simulink library "Renault_Library"  (Simulink 8.2)
settings: Solver=ode3  StartTime=0.0  StopTime=10.0  FixedStep=0.020  RelTol=1e-3
undefined symbols (not defined in the file or in its folder): ABC, AssystemProposal, b, chkval, CoeffA_C, CoeffB_C, CoeffMean_C, ConfTime_C, DiagDelay_C, DividePercent, FailureConfActive_C, FallingEdgeDuration_C, HighLevelThresholdVoltage_C, HighLevelVoltage_C, HighLevelVoltageTunable_C, InitValue_C, InputVoltRangeMax_C, InputVoltRangeMin_C, LowActiveContact_C, LowLevelThresholdVoltage_C, LowLevelVoltage_C, MaxOperatingVoltage_C, MinOperatingVoltage_C, mV2V_conversion, OUT1, OutOfRangeHigh_C, OutOfRangeLow_C, PinSupplySaturationMax, PinSupplySaturationMin, Res1Value_C, Res2Value_C, Res3Value_C, RisingEdgeDuration_C, RSA_CANSignalFailMonitoring, SampleTime_C, service14Table, service19Table, service22Table, service24Table, service28Table, service2ETable, service2FTable, service31Table, service87Table, SizeXStateDID2FTable, SizeXTable14, SizeXTable19, SizeXTable22, SizeXTable24, SizeXTable28, SizeXTable2E, SizeXTable2F, SizeXTable31, SizeXTable87, SizeYStateDID2FTable, SizeYTable14, SizeYTable19, SizeYTable22, SizeYTable24, SizeYTable28 ... (+22)
undefined symbols (not defined in the file): BatteryVoltage_ActivationThreshold_C, FrameStatus_AbsentValue_C, FrameStatus_ConfAbsentValue_C, MemThreshold_C, NAMEOFTHEMODEL_SAMPLE_TIME_CST, NbAbs1_C, NbConfAbs1_C, NbConfPres1_C, NbPres1_C, NOMDUSIGNAL_FaultConfTime_C, NOMDUSIGNAL_FaultRehabTime_C, NOMDUSIGNAL_switchvalue_C, NOMDUSIGNAL_unavailablevalue_C, PeriodFrame1_C, ResetTime_C, WakeUpTime_C
libraries: Renault_Library x112, simulink x75
(part 22 of 32)

== system Renault_Library/Services ECU/Diag/RSA_DiagnosticManagement_v02.00/DIAGNOSTIC_SANCTION_TOP/Service19/SubFunction_Supported_Service19 ==
  DIAG_BUFFER_REQUEST : Inport
  BitwiseOperator1 : simulink/Logic and Bit Operations/Bitwise Operator  logicop=AND  UseBitMask=on  BitMask=bin2dec('01111111')  BitMaskRealWorld=Stored Integer
  BitwiseOperator4 : simulink/Logic and Bit Operations/Bitwise Operator  logicop=AND  UseBitMask=on  BitMask=bin2dec('10000000')  BitMaskRealWorld=Stored Integer
  Constant : Constant  OutDataTypeStr=Inherit: Inherit via back propagation  SampleTime=-1
  Constant1 : Constant  Value=6  OutDataTypeStr=Inherit: Inherit via back propagation  SampleTime=-1
  Constant10 : Constant  Value=ReporDTCSnapshotIdentification  OutDataTypeStr=Inherit: Inherit via back propagation  SampleTime=-1
  Constant{5,9,11,13,18,20,23,28,30,34,36,40,56,58,60,62,65,69..74} (23 alike) : Constant  Value=true  OutDataTypeStr=boolean  SampleTime=-1
  Constant12 : Constant  Value=ReportDTCStoredDataByRecordNumber  OutDataTypeStr=Inherit: Inherit via back propagation  SampleTime=-1
  Constant14 : Constant  Value=5  OutDataTypeStr=Inherit: Inherit via back propagation  SampleTime=-1
  Constant15 : Constant  Value=ReportSupportedDTC  OutDataTypeStr=Inherit: Inherit via back propagation  SampleTime=-1
  Constant16 : Constant  OutDataTypeStr=Inherit: Inherit via back propagation  SampleTime=-1
  Constant17 : Constant  Value=ReporDTCSnapshotRecordByDTCNumber  OutDataTypeStr=Inherit: Inherit via back propagation  SampleTime=-1
  Constant19 : Constant  Value=4  OutDataTypeStr=Inherit: Inherit via back propagation  SampleTime=-1
  Constant2 : Constant  Value=2  OutDataTypeStr=Inherit: Inherit via back propagation  SampleTime=-1
  Constant21 : Constant  Value=10  OutDataTypeStr=Inherit: Inherit via back propagation  SampleTime=-1
  Constant22 : Constant  Value=ReportSeverityInformationOfDTC  OutDataTypeStr=Inherit: Inherit via back propagation  SampleTime=-1
  Constant24 : Constant  Value=9  OutDataTypeStr=Inherit: Inherit via back propagation  SampleTime=-1
  Constant25 : Constant  Value=7  OutDataTypeStr=Inherit: Inherit via back propagation  SampleTime=-1
  Constant26 : Constant  Value=ReportDTCExtendedDataRecordByDTCNumber  OutDataTypeStr=Inherit: Inherit via back propagation  SampleTime=-1
  Constant27 : Constant  Value=8  OutDataTypeStr=Inherit: Inherit via back propagation  SampleTime=-1
  Constant29 : Constant  Value=ReportNumberOfDTCBySeverityMaskRecord  OutDataTypeStr=Inherit: Inherit via back propagation  SampleTime=-1
  Constant3 : Constant  Value=ReportNumberOfDTCByStatusMask  OutDataTypeStr=Inherit: Inherit via back propagation  SampleTime=-1
  Constant31 : Constant  Value=11  OutDataTypeStr=Inherit: Inherit via back propagation  SampleTime=-1
  Constant32 : Constant  Value=16  OutDataTypeStr=Inherit: Inherit via back propagation  SampleTime=-1
  Constant33 : Constant  Value=ReportMostRecentTestFailedDTC  OutDataTypeStr=Inherit: Inherit via back propagation  SampleTime=-1
  Constant35 : Constant  Value=ReportMirrorMemoryDTCByStatusMask  OutDataTypeStr=Inherit: Inherit via back propagation  SampleTime=-1
  Constant37 : Constant  Value=15  OutDataTypeStr=Inherit: Inherit via back propagation  SampleTime=-1
  Constant38 : Constant  Value=ReportDTCFaultDetectionCounter  OutDataTypeStr=Inherit: Inherit via back propagation  SampleTime=-1
  Constant39 : Constant  Value=ReportMostRecentConfimedDTC  OutDataTypeStr=Inherit: Inherit via back propagation  SampleTime=-1
  Constant4 : Constant  Value=3  OutDataTypeStr=Inherit: Inherit via back propagation  SampleTime=-1
  Constant41 : Constant  Value=14  OutDataTypeStr=Inherit: Inherit via back propagation  SampleTime=-1
  Constant42 : Constant  Value=12  OutDataTypeStr=Inherit: Inherit via back propagation  SampleTime=-1
  Constant44 : Constant  Value=20  OutDataTypeStr=Inherit: Inherit via back propagation  SampleTime=-1
  Constant45 : Constant  Value=ReportEmissionsRelatedOBDDTCByStatusMask  OutDataTypeStr=Inherit: Inherit via back propagation  SampleTime=-1
  Constant47 : Constant  Value=19  OutDataTypeStr=Inherit: Inherit via back propagation  SampleTime=-1
  Constant48 : Constant  Value=17  OutDataTypeStr=Inherit: Inherit via back propagation  SampleTime=-1
  Constant49 : Constant  Value=ReportMirrorMemoryDTCExtendedDataRecordByDTCNumber  OutDataTypeStr=Inherit: Inherit via back propagation  SampleTime=-1
  Constant50 : Constant  Value=18  OutDataTypeStr=Inherit: Inherit via back propagation  SampleTime=-1
  Constant52 : Constant  Value=ReportNumberOfMirrorMemoryDTCByStatusMask  OutDataTypeStr=Inherit: Inherit via back propagation  SampleTime=-1
  Constant53 : Constant  Value=ReportFirstTestFailedDTC  OutDataTypeStr=Inherit: Inherit via back propagation  SampleTime=-1
  Constant55 : Constant  Value=13  OutDataTypeStr=Inherit: Inherit via back propagation  SampleTime=-1
  Constant57 : Constant  Value=ReportNumberOfEmissionsRelatedOBDDTCByStatusMask  OutDataTypeStr=Inherit: Inherit via back propagation  SampleTime=-1
  Constant59 : Constant  Value=ReportFirstConfirmedDTC  OutDataTypeStr=Inherit: Inherit via back propagation  SampleTime=-1
  Constant6 : Constant  Value=ReportDTCBySeverityMaskRecord  OutDataTypeStr=Inherit: Inherit via back propagation  SampleTime=-1
  Constant61 : Constant  Value=ReportWWHOBDDTCWithPermanentStatus  OutDataTypeStr=Inherit: Inherit via back propagation  SampleTime=-1
  Constant63 : Constant  Value=85  OutDataTypeStr=Inherit: Inherit via back propagation  SampleTime=-1
  Constant64 : Constant  Value=ReportWWHOBDDTCByMaskRecord  OutDataTypeStr=Inherit: Inherit via back propagation  SampleTime=-1
  Constant66 : Constant  Value=66  OutDataTypeStr=Inherit: Inherit via back propagation  SampleTime=-1
  Constant67 : Constant  Value=21  OutDataTypeStr=Inherit: Inherit via back propagation  SampleTime=-1
  Constant68 : Constant  Value=ReportDTCWithPermanetStatus  OutDataTypeStr=Inherit: Inherit via back propagation  SampleTime=-1
  Constant8 : Constant  Value=ReportDTCByStatusMask  OutDataTypeStr=Inherit: Inherit via back propagation  SampleTime=-1
  LogicalOperator{,1..3,5..23} (23 alike) : Logic  OutDataTypeStr=boolean
  LogicalOperator4 : Logic  Operator=OR  Inputs=23  OutDataTypeStr=boolean
  RelationalOperator{,1..46} (47 alike) : RelationalOperator  Operator===  OutDataTypeStr=boolean  InputSameDT=on
  Selector : Selector  InputPortWidth=4095  IndexOptions=Index vector (dialog)  Indices=[2]  OutputSizes=1
  ShiftArithmetic3 : ArithShift  BitShiftNumber=7
  SUBFUNCTION_SUPPORTED : Outport
  SUPPRESS_POS_RES : Outport  Port=2
  wires:
  DIAG_BUFFER_REQUEST/1 -> Selector/1
  Constant/1 -> RelationalOperator/2
  Constant2/1 -> RelationalOperator2/2
  Constant4/1 -> RelationalOperator3/2
  Constant3/1 -> RelationalOperator4/1
  Constant5/1 -> RelationalOperator4/2
  RelationalOperator/1 -> LogicalOperator/2
  RelationalOperator4/1 -> LogicalOperator/1
  Constant36/1 -> RelationalOperator6/2
  Constant8/1 -> RelationalOperator6/1
  RelationalOperator2/1 -> LogicalOperator2/2
  RelationalOperator6/1 -> LogicalOperator2/1
  Constant10/1 -> RelationalOperator7/1
  Constant56/1 -> RelationalOperator7/2
  RelationalOperator7/1 -> LogicalOperator3/1
  RelationalOperator3/1 -> LogicalOperator3/2
  RelationalOperator16/1 -> SUPPRESS_POS_RES/1
  BitwiseOperator4/1 -> ShiftArithmetic3/1
  ShiftArithmetic3/1 -> RelationalOperator16/1
  Selector/1 -> BitwiseOperator4/1, BitwiseOperator1/1
  Constant16/1 -> RelationalOperator16/2
  BitwiseOperator1/1 -> RelationalOperator/1, RelationalOperator2/1, RelationalOperator3/1, RelationalOperator12/1, RelationalOperator8/1, RelationalOperator1/1, RelationalOperator11/1, RelationalOperator14/1, RelationalOperator5/1, RelationalOperator19/1, RelationalOperator21/1, RelationalOperator32/1, RelationalOperator34/1, RelationalOperator25/1, RelationalOperator39/1, RelationalOperator22/1, RelationalOperator24/1, RelationalOperator27/1, RelationalOperator36/1, RelationalOperator31/1, RelationalOperator42/1, RelationalOperator46/1, RelationalOperator44/1
  Constant19/1 -> RelationalOperator12/2
  Constant17/1 -> RelationalOperator13/1
  Constant58/1 -> RelationalOperator13/2
  RelationalOperator13/1 -> LogicalOperator6/1
  RelationalOperator12/1 -> LogicalOperator6/2
  Constant14/1 -> RelationalOperator8/2
  Constant12/1 -> RelationalOperator9/1
  Constant71/1 -> RelationalOperator9/2
  RelationalOperator9/1 -> LogicalOperator5/1
  RelationalOperator8/1 -> LogicalOperator5/2
  Constant1/1 -> RelationalOperator1/2
  Constant25/1 -> RelationalOperator11/2
  Constant27/1 -> RelationalOperator14/2
  Constant60/1 -> RelationalOperator15/2
  RelationalOperator1/1 -> LogicalOperator1/2
  RelationalOperator15/1 -> LogicalOperator1/1
  Constant62/1 -> RelationalOperator17/2
  Constant29/1 -> RelationalOperator17/1
  RelationalOperator11/1 -> LogicalOperator7/2
  RelationalOperator17/1 -> LogicalOperator7/1
  Constant6/1 -> RelationalOperator18/1
  Constant65/1 -> RelationalOperator18/2
  RelationalOperator18/1 -> LogicalOperator8/1
  RelationalOperator14/1 -> LogicalOperator8/2
  Constant24/1 -> RelationalOperator5/2
  Constant22/1 -> RelationalOperator10/1
  Constant69/1 -> RelationalOperator10/2
  RelationalOperator10/1 -> LogicalOperator10/1
  RelationalOperator5/1 -> LogicalOperator10/2
  Constant21/1 -> RelationalOperator19/2
  Constant15/1 -> RelationalOperator20/1
  Constant70/1 -> RelationalOperator20/2
  RelationalOperator20/1 -> LogicalOperator9/1
  RelationalOperator19/1 -> LogicalOperator9/2
  Constant31/1 -> RelationalOperator21/2
  Constant42/1 -> RelationalOperator32/2
  Constant55/1 -> RelationalOperator34/2
  Constant53/1 -> RelationalOperator35/1
  Constant72/1 -> RelationalOperator35/2
  RelationalOperator21/1 -> LogicalOperator11/2
  RelationalOperator35/1 -> LogicalOperator11/1
  Constant73/1 -> RelationalOperator37/2
  Constant59/1 -> RelationalOperator37/1
  RelationalOperator32/1 -> LogicalOperator14/2
  RelationalOperator37/1 -> LogicalOperator14/1
  Constant33/1 -> RelationalOperator38/1
  Constant11/1 -> RelationalOperator38/2
  RelationalOperator38/1 -> LogicalOperator15/1
  RelationalOperator34/1 -> LogicalOperator15/2
  Constant41/1 -> RelationalOperator25/2
  Constant39/1 -> RelationalOperator26/1
  Constant9/1 -> RelationalOperator26/2
  RelationalOperator26/1 -> LogicalOperator17/1
  RelationalOperator25/1 -> LogicalOperator17/2
  Constant37/1 -> RelationalOperator39/2
  Constant35/1 -> RelationalOperator40/1
  Constant13/1 -> RelationalOperator40/2
  RelationalOperator40/1 -> LogicalOperator16/1
  RelationalOperator39/1 -> LogicalOperator16/2
  Constant32/1 -> RelationalOperator22/2
  Constant48/1 -> RelationalOperator24/2
  Constant50/1 -> RelationalOperator27/2
  Constant18/1 -> RelationalOperator28/2
  RelationalOperator22/1 -> LogicalOperator12/2
  RelationalOperator28/1 -> LogicalOperator12/1
  Constant20/1 -> RelationalOperator29/2
  Constant52/1 -> RelationalOperator29/1
  RelationalOperator24/1 -> LogicalOperator18/2
  RelationalOperator29/1 -> LogicalOperator18/1
  Constant57/1 -> RelationalOperator30/1
  Constant23/1 -> RelationalOperator30/2
  RelationalOperator30/1 -> LogicalOperator19/1
  RelationalOperator27/1 -> LogicalOperator19/2
  Constant47/1 -> RelationalOperator36/2
  Constant45/1 -> RelationalOperator23/1
  Constant74/1 -> RelationalOperator23/2
  RelationalOperator23/1 -> LogicalOperator13/1
  RelationalOperator36/1 -> LogicalOperator13/2
  Constant38/1 -> RelationalOperator33/1
  Constant28/1 -> RelationalOperator33/2
  RelationalOperator33/1 -> LogicalOperator20/1
  RelationalOperator31/1 -> LogicalOperator20/2
  Constant44/1 -> RelationalOperator31/2
  Constant67/1 -> RelationalOperator42/2
  Constant68/1 -> RelationalOperator43/1
  Constant30/1 -> RelationalOperator43/2
  RelationalOperator43/1 -> LogicalOperator22/1
  RelationalOperator42/1 -> LogicalOperator22/2
  Constant66/1 -> RelationalOperator46/2
  Constant64/1 -> RelationalOperator41/1
  Constant34/1 -> RelationalOperator41/2
  RelationalOperator41/1 -> LogicalOperator21/1
  RelationalOperator46/1 -> LogicalOperator21/2
  Constant61/1 -> RelationalOperator45/1
  Constant40/1 -> RelationalOperator45/2
  RelationalOperator45/1 -> LogicalOperator23/1
  RelationalOperator44/1 -> LogicalOperator23/2
  Constant63/1 -> RelationalOperator44/2
  LogicalOperator/1 -> LogicalOperator4/1
  LogicalOperator2/1 -> LogicalOperator4/2
  LogicalOperator3/1 -> LogicalOperator4/3
  LogicalOperator6/1 -> LogicalOperator4/4
  LogicalOperator5/1 -> LogicalOperator4/5
  LogicalOperator1/1 -> LogicalOperator4/6
  LogicalOperator7/1 -> LogicalOperator4/7
  LogicalOperator8/1 -> LogicalOperator4/8
  LogicalOperator10/1 -> LogicalOperator4/9
  LogicalOperator9/1 -> LogicalOperator4/10
  LogicalOperator11/1 -> LogicalOperator4/11
  LogicalOperator14/1 -> LogicalOperator4/12
  LogicalOperator15/1 -> LogicalOperator4/13
  LogicalOperator17/1 -> LogicalOperator4/14
  LogicalOperator16/1 -> LogicalOperator4/15
  LogicalOperator12/1 -> LogicalOperator4/16
  LogicalOperator18/1 -> LogicalOperator4/17
  LogicalOperator19/1 -> LogicalOperator4/18
  LogicalOperator13/1 -> LogicalOperator4/19
  LogicalOperator20/1 -> LogicalOperator4/20
  LogicalOperator22/1 -> LogicalOperator4/21
  LogicalOperator21/1 -> LogicalOperator4/22
  LogicalOperator23/1 -> LogicalOperator4/23
  Constant26/1 -> RelationalOperator15/1
  Constant49/1 -> RelationalOperator28/1
  LogicalOperator4/1 -> SUBFUNCTION_SUPPORTED/1

== system Renault_Library/Services ECU/Diag/RSA_DiagnosticManagement_v02.00/DIAGNOSTIC_SANCTION_TOP/Service22/Check_Lenght_Message_Response_Service22 ==
  RESPONSE_SIZE : Inport
  Constant8 : Constant  Value=4094  OutDataTypeStr=Inherit: Inherit via back propagation  SampleTime=-1
  RelationalOperator9 : RelationalOperator  Operator=<  OutDataTypeStr=boolean  InputSameDT=on
  LENGTH_RESPONSE_CORRECT : Outport
  wires:
  RESPONSE_SIZE/1 -> RelationalOperator9/1
  Constant8/1 -> RelationalOperator9/2
  RelationalOperator9/1 -> LENGTH_RESPONSE_CORRECT/1

== system Renault_Library/Services ECU/Diag/RSA_DiagnosticManagement_v02.00/DIAGNOSTIC_SANCTION_TOP/Service22/Check_Lenght_Message_Service22 ==
  REQ_DATA_NUMBER : Inport
  Constant1 : Constant  Value=3  OutDataTypeStr=Inherit: Inherit via back propagation  SampleTime=-1
  Constant8 : Constant  Value=4094  OutDataTypeStr=Inherit: Inherit via back propagation  SampleTime=-1
  LogicalOperator : Logic  OutDataTypeStr=boolean
  RelationalOperator1 : RelationalOperator  OutDataTypeStr=boolean  InputSameDT=on
  RelationalOperator9 : RelationalOperator  Operator=<  OutDataTypeStr=boolean  InputSameDT=on
  LENGTH_MESSAGE_CORRECT : Outport
  wires:
  REQ_DATA_NUMBER/1 -> RelationalOperator9/1, RelationalOperator1/1
  Constant8/1 -> RelationalOperator9/2
  Constant1/1 -> RelationalOperator1/2
  RelationalOperator9/1 -> LogicalOperator/1
  RelationalOperator1/1 -> LogicalOperator/2
  LogicalOperator/1 -> LENGTH_MESSAGE_CORRECT/1

== system Renault_Library/Services ECU/Diag/RSA_DiagnosticManagement_v02.00/DIAGNOSTIC_SANCTION_TOP/Service22/Parameter_Valid_Service22 ==
  REQ_DATA_NUMBER : Inport
  DIAG_BUFFER_REQUEST : Inport  Port=2
  SESSION_ACTIVE : Inport  Port=3
  Parameter_service22 : Stateflow chart  TreatAsAtomicUnit=on
  RESPONSE_SIZE : Outport
  SECURITY_ACCESS_ACCEPTED : Outport  Port=2
  PARAMETER_VALID : Outport  Port=3
  LIST_DID_SERVICE_22 : Outport  Port=4  PortDimensions=[2046 3]
  wires:
  DIAG_BUFFER_REQUEST/1 -> Parameter_service22/1(DIAG_BUFFER_REQUEST)
  REQ_DATA_NUMBER/1 -> Parameter_service22/2(REQ_DATA_NUMBER)
  Parameter_service22/1(PARAMETER_VALID) -> PARAMETER_VALID/1
  Parameter_service22/2(LIST_DID_SERVICE_22) -> LIST_DID_SERVICE_22/1
  Parameter_service22/3(RESPONSE_SIZE) -> RESPONSE_SIZE/1
  Parameter_service22/4(SECURITY_ACCESS_ACCEPTED) -> SECURITY_ACCESS_ACCEPTED/1
  SESSION_ACTIVE/1 -> Parameter_service22/3(SESSION_ACTIVE)

== system Renault_Library/Services ECU/Diag/RSA_DiagnosticManagement_v02.00/DIAGNOSTIC_SANCTION_TOP/Service23/Check_Lenght_Message_Service23 ==
  REQ_DATA_NUMBER : Inport
  Constant1 : Constant  Value=4  OutDataTypeStr=Inherit: Inherit via back propagation  SampleTime=-1
  Constant8 : Constant  Value=4094  OutDataTypeStr=Inherit: Inherit via back propagation  SampleTime=-1
  LogicalOperator : Logic  OutDataTypeStr=boolean
  RelationalOperator1 : RelationalOperator  OutDataTypeStr=boolean  InputSameDT=on
  RelationalOperator9 : RelationalOperator  Operator=<  OutDataTypeStr=boolean  InputSameDT=on
  LENGTH_MESSAGE_CORRECT : Outport
  wires:
  REQ_DATA_NUMBER/1 -> RelationalOperator9/1, RelationalOperator1/1
  Constant8/1 -> RelationalOperator9/2
  Constant1/1 -> RelationalOperator1/2
  RelationalOperator9/1 -> LogicalOperator/1
  RelationalOperator1/1 -> LogicalOperator/2
  LogicalOperator/1 -> LENGTH_MESSAGE_CORRECT/1

== system Renault_Library/Services ECU/Diag/RSA_DiagnosticManagement_v02.00/DIAGNOSTIC_SANCTION_TOP/Service23/Parameter_Valid_Service23 ==
  REQ_DATA_NUMBER : Inport
  DIAG_BUFFER_REQUEST : Inport  Port=2
  SESSION_ACTIVE : Inport  Port=3
  Parameter_service23 : Stateflow chart  TreatAsAtomicUnit=on
  SECURITY_ACCESS_ACCEPTED : Outport
  PARAMETER_VALID : Outport  Port=2
  wires:
  DIAG_BUFFER_REQUEST/1 -> Parameter_service23/1(DIAG_BUFFER_REQUEST)
  REQ_DATA_NUMBER/1 -> Parameter_service23/2(REQ_DATA_NUMBER)
  Parameter_service23/1(PARAMETER_VALID) -> PARAMETER_VALID/1
  Parameter_service23/2(SECURITY_ACCESS_ACCEPTED) -> SECURITY_ACCESS_ACCEPTED/1
  SESSION_ACTIVE/1 -> Parameter_service23/3(SESSION_ACTIVE)

== system Renault_Library/Services ECU/Diag/RSA_DiagnosticManagement_v02.00/DIAGNOSTIC_SANCTION_TOP/Service24/Check_Lenght_Message_Service24 ==
  REQ_DATA_NUMBER : Inport
  Constant8 : Constant  Value=3  OutDataTypeStr=Inherit: Inherit via back propagation  SampleTime=-1
  RelationalOperator9 : RelationalOperator  Operator===  OutDataTypeStr=boolean  InputSameDT=on
  LENGTH_MESSAGE_CORRECT : Outport
  wires:
  REQ_DATA_NUMBER/1 -> RelationalOperator9/1
  Constant8/1 -> RelationalOperator9/2
  RelationalOperator9/1 -> LENGTH_MESSAGE_CORRECT/1

== system Renault_Library/Services ECU/Diag/RSA_DiagnosticManagement_v02.00/DIAGNOSTIC_SANCTION_TOP/Service24/Parameter_Valid_Service24 ==
  DIAG_BUFFER_REQUEST : Inport
  SESSION_ACTIVE : Inport  Port=2
  Parameter_service24 : Stateflow chart  TreatAsAtomicUnit=on
  SECURITY_ACCESS_ACCEPTED : Outport
  PARAMETER_VALID : Outport  Port=2
  wires:
  DIAG_BUFFER_REQUEST/1 -> Parameter_service24/1(DIAG_BUFFER_REQUEST)
  Parameter_service24/1(PARAMETER_VALID) -> PARAMETER_VALID/1
  Parameter_service24/2(SECURITY_ACCESS_ACCEPTED) -> SECURITY_ACCESS_ACCEPTED/1
  SESSION_ACTIVE/1 -> Parameter_service24/2(SESSION_ACTIVE)

== system Renault_Library/Services ECU/Diag/RSA_DiagnosticManagement_v02.00/DIAGNOSTIC_SANCTION_TOP/Service27/Check_Lenght_Message_Service27 ==
  REQ_DATA_NUMBER : Inport
  Constant1 : Constant  Value=2  OutDataTypeStr=Inherit: Inherit via back propagation  SampleTime=-1
  Constant8 : Constant  Value=4094  OutDataTypeStr=Inherit: Inherit via back propagation  SampleTime=-1
  LogicalOperator : Logic  OutDataTypeStr=boolean
  RelationalOperator1 : RelationalOperator  OutDataTypeStr=boolean  InputSameDT=on
  RelationalOperator9 : RelationalOperator  Operator=<  OutDataTypeStr=boolean  InputSameDT=on
  LENGTH_MESSAGE_CORRECT : Outport
  wires:
  REQ_DATA_NUMBER/1 -> RelationalOperator9/1, RelationalOperator1/1
  Constant8/1 -> RelationalOperator9/2
  Constant1/1 -> RelationalOperator1/2
  RelationalOperator9/1 -> LogicalOperator/1
  RelationalOperator1/1 -> LogicalOperator/2
  LogicalOperator/1 -> LENGTH_MESSAGE_CORRECT/1

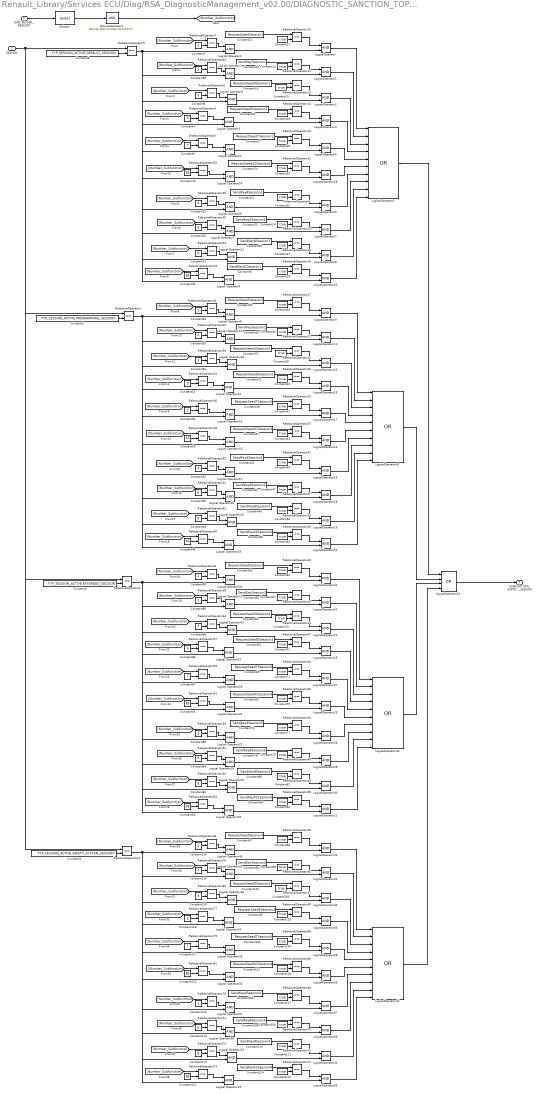
== system Renault_Library/Services ECU/Diag/RSA_DiagnosticManagement_v02.00/DIAGNOSTIC_SANCTION_TOP/Service27/SubFunction_In_Active_Session_Service27 ==
  SESSION : Inport
  DIAG_BUFFER_REQUEST : Inport  Port=2
  BitwiseOperator1 : simulink/Logic and Bit Operations/Bitwise Operator  logicop=AND  UseBitMask=on  BitMask=bin2dec('01111111')  BitMaskRealWorld=Stored Integer
  Constant1 : Constant  Value=TYP_SESSION_ACTIVE.PROGRAMMING_SESSION  OutDataTypeStr=Inherit: Inherit via back propagation  SampleTime=-1
  Constant10 : Constant  Value=RequestSeed5Session1  OutDataTypeStr=Inherit: Inherit via back propagation  SampleTime=-1
  Constant{11,13,15,18..20,22,24,27..28,34,36,39..41,43,45,47,58,62,64,67,70..72,75,77,79,81,92,96,98,100,103..105,107,109,111,122} (40 alike) : Constant  Value=true  OutDataTypeStr=boolean  SampleTime=-1
  Constant101 : Constant  Value=RequestSeed3Session4  OutDataTypeStr=Inherit: Inherit via back propagation  SampleTime=-1
  Constant102 : Constant  Value=RequestSeed7Session4  OutDataTypeStr=Inherit: Inherit via back propagation  SampleTime=-1
  Constant106 : Constant  Value=SendKey4Session4  OutDataTypeStr=Inherit: Inherit via back propagation  SampleTime=-1
  Constant108 : Constant  Value=SendKey6Session4  OutDataTypeStr=Inherit: Inherit via back propagation  SampleTime=-1
  Constant110 : Constant  Value=SendKey8Session4  OutDataTypeStr=Inherit: Inherit via back propagation  SampleTime=-1
  Constant{29,48,82,112} (4 alike) : Constant  Value=66  OutDataTypeStr=Inherit: Inherit via back propagation  SampleTime=-1
  Constant{31,49,83,113} (4 alike) : Constant  Value=8  OutDataTypeStr=Inherit: Inherit via back propagation  SampleTime=-1
  Constant{32,50,84,114} (4 alike) : Constant  Value=6  OutDataTypeStr=Inherit: Inherit via back propagation  SampleTime=-1
  Constant{33,51,85,115} (4 alike) : Constant  Value=4  OutDataTypeStr=Inherit: Inherit via back propagation  SampleTime=-1
  Constant{4,52,86,116} (4 alike) : Constant  Value=5  OutDataTypeStr=Inherit: Inherit via back propagation  SampleTime=-1
  Constant{5,53,87,117} (4 alike) : Constant  Value=7  OutDataTypeStr=Inherit: Inherit via back propagation  SampleTime=-1
  Constant{6,54,88,118} (4 alike) : Constant  Value=3  OutDataTypeStr=Inherit: Inherit via back propagation  SampleTime=-1
  Constant{55,65,89,119} (4 alike) : Constant  Value=2  OutDataTypeStr=Inherit: Inherit via back propagation  SampleTime=-1
  Constant12 : Constant  Value=RequestSeedSession1  OutDataTypeStr=Inherit: Inherit via back propagation  SampleTime=-1
  Constant{7,56,90,120} (4 alike) : Constant  OutDataTypeStr=Inherit: Inherit via back propagation  SampleTime=-1
  Constant121 : Constant  Value=RequestSeed41Session4  OutDataTypeStr=Inherit: Inherit via back propagation  SampleTime=-1
  Constant{59,76,93,123} (4 alike) : Constant  Value=65  OutDataTypeStr=Inherit: Inherit via back propagation  SampleTime=-1
  Constant124 : Constant  Value=SendKey42Session4  OutDataTypeStr=Inherit: Inherit via back propagation  SampleTime=-1
  Constant14 : Constant  Value=SendKeySession1  OutDataTypeStr=Inherit: Inherit via back propagation  SampleTime=-1
  Constant16 : Constant  Value=RequestSeed3Session1  OutDataTypeStr=Inherit: Inherit via back propagation  SampleTime=-1
  Constant17 : Constant  Value=RequestSeed7Session1  OutDataTypeStr=Inherit: Inherit via back propagation  SampleTime=-1
  Constant2 : Constant  Value=TYP_SESSION_ACTIVE.EXTENDED_SESSION  OutDataTypeStr=Inherit: Inherit via back propagation  SampleTime=-1
  Constant21 : Constant  Value=SendKey4Session1  OutDataTypeStr=Inherit: Inherit via back propagation  SampleTime=-1
  Constant23 : Constant  Value=SendKey6Session1  OutDataTypeStr=Inherit: Inherit via back propagation  SampleTime=-1
  Constant25 : Constant  Value=SendKey8Session1  OutDataTypeStr=Inherit: Inherit via back propagation  SampleTime=-1
  Constant26 : Constant  Value=RequestSeed5Session2  OutDataTypeStr=Inherit: Inherit via back propagation  SampleTime=-1
  Constant3 : Constant  Value=TYP_SESSION_ACTIVE.SAFETY_SYSTEM_SESSION  OutDataTypeStr=Inherit: Inherit via back propagation  SampleTime=-1
  Constant30 : Constant  Value=RequestSeedSession2  OutDataTypeStr=Inherit: Inherit via back propagation  SampleTime=-1
  Constant35 : Constant  Value=SendKeySession2  OutDataTypeStr=Inherit: Inherit via back propagation  SampleTime=-1
  Constant37 : Constant  Value=RequestSeed3Session2  OutDataTypeStr=Inherit: Inherit via back propagation  SampleTime=-1
  Constant38 : Constant  Value=RequestSeed7Session2  OutDataTypeStr=Inherit: Inherit via back propagation  SampleTime=-1
  Constant42 : Constant  Value=SendKey4Session2  OutDataTypeStr=Inherit: Inherit via back propagation  SampleTime=-1
  Constant44 : Constant  Value=SendKey6Session2  OutDataTypeStr=Inherit: Inherit via back propagation  SampleTime=-1
  Constant46 : Constant  Value=SendKey8Session2  OutDataTypeStr=Inherit: Inherit via back propagation  SampleTime=-1
  Constant57 : Constant  Value=RequestSeed41Session2  OutDataTypeStr=Inherit: Inherit via back propagation  SampleTime=-1
  Constant60 : Constant  Value=SendKey42Session2  OutDataTypeStr=Inherit: Inherit via back propagation  SampleTime=-1
  Constant61 : Constant  Value=RequestSeed5Session3  OutDataTypeStr=Inherit: Inherit via back propagation  SampleTime=-1
  Constant63 : Constant  Value=RequestSeedSession3  OutDataTypeStr=Inherit: Inherit via back propagation  SampleTime=-1
  Constant66 : Constant  Value=SendKeySession3  OutDataTypeStr=Inherit: Inherit via back propagation  SampleTime=-1
  Constant68 : Constant  Value=RequestSeed3Session3  OutDataTypeStr=Inherit: Inherit via back propagation  SampleTime=-1
  Constant69 : Constant  Value=RequestSeed7Session3  OutDataTypeStr=Inherit: Inherit via back propagation  SampleTime=-1
  Constant73 : Constant  Value=SendKey4Session3  OutDataTypeStr=Inherit: Inherit via back propagation  SampleTime=-1
  Constant74 : Constant  Value=RequestSeed41Session1  OutDataTypeStr=Inherit: Inherit via back propagation  SampleTime=-1
  Constant78 : Constant  Value=SendKey6Session3  OutDataTypeStr=Inherit: Inherit via back propagation  SampleTime=-1
  Constant8 : Constant  Value=TYP_SESSION_ACTIVE.DEFAULT_SESSION  OutDataTypeStr=Inherit: Inherit via back propagation  SampleTime=-1
  Constant80 : Constant  Value=SendKey8Session3  OutDataTypeStr=Inherit: Inherit via back propagation  SampleTime=-1
  Constant9 : Constant  Value=SendKey42Session1  OutDataTypeStr=Inherit: Inherit via back propagation  SampleTime=-1
  Constant91 : Constant  Value=RequestSeed41Session3  OutDataTypeStr=Inherit: Inherit via back propagation  SampleTime=-1
  Constant94 : Constant  Value=SendKey42Session3  OutDataTypeStr=Inherit: Inherit via back propagation  SampleTime=-1
  Constant95 : Constant  Value=RequestSeed5Session4  OutDataTypeStr=Inherit: Inherit via back propagation  SampleTime=-1
  Constant97 : Constant  Value=RequestSeedSession4  OutDataTypeStr=Inherit: Inherit via back propagation  SampleTime=-1
  Constant99 : Constant  Value=SendKeySession4  OutDataTypeStr=Inherit: Inherit via back propagation  SampleTime=-1
  Logical Operator{1..40} (40 alike) : Logic  OutDataTypeStr=boolean
  LogicalOperator{,1..3,5..23,25..41} (40 alike) : Logic  OutDataTypeStr=boolean
  LogicalOperator24 : Logic  Operator=OR  Inputs=4  OutDataTypeStr=boolean
  LogicalOperator{4,42..44} (4 alike) : Logic  Operator=OR  Inputs=10  OutDataTypeStr=boolean
  RelationalOperator{1..84} (84 alike) : RelationalOperator  Operator===  OutDataTypeStr=boolean  InputSameDT=on
  Selector : Selector  InputPortWidth=4095  IndexOptions=Index vector (dialog)  Indices=[2]  OutputSizes=1
  SUBFUNCTION_SUPPORTED_IN_ACTIVE_SESSION : Outport
  wires:
  Constant8/1 -> RelationalOperator9/2
  SESSION/1 -> RelationalOperator9/1, RelationalOperator1/1, RelationalOperator2/1, RelationalOperator3/1
  Constant1/1 -> RelationalOperator1/2
  Constant2/1 -> RelationalOperator2/2
  Constant3/1 -> RelationalOperator3/2
  Selector/1 -> BitwiseOperator1/1
  DIAG_BUFFER_REQUEST/1 -> Selector/1
  Constant7/1 -> RelationalOperator7/2
  Constant12/1 -> RelationalOperator10/1
  Constant11/1 -> RelationalOperator10/2
  RelationalOperator10/1 -> LogicalOperator/1
  Constant13/1 -> RelationalOperator11/2
  Constant14/1 -> RelationalOperator11/1
  RelationalOperator11/1 -> LogicalOperator1/1
  Constant15/1 -> RelationalOperator12/2
  Constant16/1 -> RelationalOperator12/1
  RelationalOperator12/1 -> LogicalOperator2/1
  Constant10/1 -> RelationalOperator14/1
  Constant18/1 -> RelationalOperator14/2
  RelationalOperator14/1 -> LogicalOperator3/1
  Constant17/1 -> RelationalOperator13/1
  Constant19/1 -> RelationalOperator13/2
  RelationalOperator13/1 -> LogicalOperator6/1
  Logical Operator2/1 -> LogicalOperator1/2
  Logical Operator1/1 -> LogicalOperator/2
  RelationalOperator9/1 -> Logical Operator1/2, Logical Operator2/2, Logical Operator3/2, Logical Operator4/2, Logical Operator5/2, Logical Operator24/2, Logical Operator6/2, Logical Operator7/2, Logical Operator11/2, Logical Operator9/2
  Constant65/1 -> RelationalOperator45/2
  RelationalOperator45/1 -> Logical Operator2/1
  Constant4/1 -> RelationalOperator4/2
  Constant5/1 -> RelationalOperator5/2
  RelationalOperator5/1 -> Logical Operator4/1
  Logical Operator4/1 -> LogicalOperator6/2
  Logical Operator3/1 -> LogicalOperator3/2
  Constant6/1 -> RelationalOperator6/2
  Logical Operator5/1 -> LogicalOperator2/2
  RelationalOperator7/1 -> Logical Operator1/1
  RelationalOperator4/1 -> Logical Operator3/1
  RelationalOperator6/1 -> Logical Operator5/1
  LogicalOperator24/1 -> SUBFUNCTION_SUPPORTED_IN_ACTIVE_SESSION/1
  BitwiseOperator1/1 -> [Number_Subfunction]
  [Number_Subfunction] -> RelationalOperator7/1
  [Number_Subfunction] -> RelationalOperator45/1
  [Number_Subfunction] -> RelationalOperator6/1
  [Number_Subfunction] -> RelationalOperator4/1
  [Number_Subfunction] -> RelationalOperator5/1
  Constant74/1 -> RelationalOperator51/1
  Constant20/1 -> RelationalOperator51/2
  RelationalOperator51/1 -> LogicalOperator28/1
  Constant76/1 -> RelationalOperator52/2
  RelationalOperator52/1 -> Logical Operator24/1
  Logical Operator24/1 -> LogicalOperator28/2
  [Number_Subfunction] -> RelationalOperator52/1
  Constant33/1 -> RelationalOperator25/2
  Constant21/1 -> RelationalOperator8/1
  Constant22/1 -> RelationalOperator8/2
  RelationalOperator8/1 -> LogicalOperator5/1
  Constant24/1 -> RelationalOperator15/2
  Constant23/1 -> RelationalOperator15/1
  RelationalOperator15/1 -> LogicalOperator7/1
  Constant27/1 -> RelationalOperator16/2
  Constant25/1 -> RelationalOperator16/1
  RelationalOperator16/1 -> LogicalOperator8/1
  Constant9/1 -> RelationalOperator18/1
  Constant28/1 -> RelationalOperator18/2
  RelationalOperator18/1 -> LogicalOperator10/1
  Logical Operator7/1 -> LogicalOperator7/2
  Logical Operator6/1 -> LogicalOperator5/2
  Constant32/1 -> RelationalOperator20/2
  RelationalOperator20/1 -> Logical Operator7/1
  Constant29/1 -> RelationalOperator19/2
  Logical Operator9/1 -> LogicalOperator10/2
  Constant31/1 -> RelationalOperator24/2
  Logical Operator11/1 -> LogicalOperator8/2
  RelationalOperator25/1 -> Logical Operator6/1
  RelationalOperator19/1 -> Logical Operator9/1
  RelationalOperator24/1 -> Logical Operator11/1
  [Number_Subfunction] -> RelationalOperator25/1
  [Number_Subfunction] -> RelationalOperator20/1
  [Number_Subfunction] -> RelationalOperator24/1
  [Number_Subfunction] -> RelationalOperator19/1
  LogicalOperator/1 -> LogicalOperator4/1
  LogicalOperator1/1 -> LogicalOperator4/2
  LogicalOperator2/1 -> LogicalOperator4/3
  LogicalOperator3/1 -> LogicalOperator4/4
  LogicalOperator6/1 -> LogicalOperator4/5
  LogicalOperator28/1 -> LogicalOperator4/6
  LogicalOperator5/1 -> LogicalOperator4/7
  LogicalOperator7/1 -> LogicalOperator4/8
  LogicalOperator8/1 -> LogicalOperator4/9
  LogicalOperator10/1 -> LogicalOperator4/10
  Constant56/1 -> RelationalOperator40/2
  Constant30/1 -> RelationalOperator17/1
  Constant34/1 -> RelationalOperator17/2
  RelationalOperator17/1 -> LogicalOperator9/1
  Constant36/1 -> RelationalOperator21/2
  Constant35/1 -> RelationalOperator21/1
  RelationalOperator21/1 -> LogicalOperator11/1
  Constant39/1 -> RelationalOperator22/2
  Constant37/1 -> RelationalOperator22/1
  RelationalOperator22/1 -> LogicalOperator13/1
  Constant26/1 -> RelationalOperator26/1
  Constant40/1 -> RelationalOperator26/2
  RelationalOperator26/1 -> LogicalOperator15/1
  Constant38/1 -> RelationalOperator23/1
  Constant41/1 -> RelationalOperator23/2
  RelationalOperator23/1 -> LogicalOperator17/1
  Logical Operator12/1 -> LogicalOperator11/2
  Logical Operator8/1 -> LogicalOperator9/2
  RelationalOperator1/1 -> Logical Operator19/2, Logical Operator10/2, Logical Operator18/2, Logical Operator17/2, Logical Operator13/2, Logical Operator16/2, Logical Operator15/2, Logical Operator14/2, Logical Operator12/2, Logical Operator8/2
  Constant55/1 -> RelationalOperator35/2
  RelationalOperator35/1 -> Logical Operator12/1
  Constant52/1 -> RelationalOperator34/2
  Constant53/1 -> RelationalOperator36/2
  RelationalOperator36/1 -> Logical Operator15/1
  Logical Operator15/1 -> LogicalOperator17/2
  Logical Operator14/1 -> LogicalOperator15/2
  Constant54/1 -> RelationalOperator39/2
  Logical Operator16/1 -> LogicalOperator13/2
  RelationalOperator40/1 -> Logical Operator8/1
  RelationalOperator34/1 -> Logical Operator14/1
  RelationalOperator39/1 -> Logical Operator16/1
  [Number_Subfunction] -> RelationalOperator40/1
  [Number_Subfunction] -> RelationalOperator35/1
  [Number_Subfunction] -> RelationalOperator39/1
  [Number_Subfunction] -> RelationalOperator34/1
  [Number_Subfunction] -> RelationalOperator36/1
  Constant57/1 -> RelationalOperator37/1
  Constant43/1 -> RelationalOperator37/2
  RelationalOperator37/1 -> LogicalOperator14/1
  Constant59/1 -> RelationalOperator38/2
  RelationalOperator38/1 -> Logical Operator13/1
  Logical Operator13/1 -> LogicalOperator14/2
  [Number_Subfunction] -> RelationalOperator38/1
  Constant51/1 -> RelationalOperator33/2
  Constant42/1 -> RelationalOperator41/1
  Constant45/1 -> RelationalOperator41/2
  RelationalOperator41/1 -> LogicalOperator16/1
  Constant47/1 -> RelationalOperator27/2
  Constant44/1 -> RelationalOperator27/1
  RelationalOperator27/1 -> LogicalOperator18/1
  Constant58/1 -> RelationalOperator28/2
  Constant46/1 -> RelationalOperator28/1
  RelationalOperator28/1 -> LogicalOperator19/1
  Constant60/1 -> RelationalOperator29/1
  Constant62/1 -> RelationalOperator29/2
  RelationalOperator29/1 -> LogicalOperator12/1
  Logical Operator18/1 -> LogicalOperator18/2
  Logical Operator17/1 -> LogicalOperator16/2
  Constant50/1 -> RelationalOperator31/2
  RelationalOperator31/1 -> Logical Operator18/1
  Constant48/1 -> RelationalOperator30/2
  Logical Operator19/1 -> LogicalOperator12/2
  Constant49/1 -> RelationalOperator32/2
  Logical Operator10/1 -> LogicalOperator19/2
  RelationalOperator33/1 -> Logical Operator17/1
  RelationalOperator30/1 -> Logical Operator19/1
  RelationalOperator32/1 -> Logical Operator10/1
  [Number_Subfunction] -> RelationalOperator33/1
  [Number_Subfunction] -> RelationalOperator31/1
  [Number_Subfunction] -> RelationalOperator32/1
  [Number_Subfunction] -> RelationalOperator30/1
  Constant90/1 -> RelationalOperator63/2
  Constant63/1 -> RelationalOperator42/1
  Constant64/1 -> RelationalOperator42/2
  RelationalOperator42/1 -> LogicalOperator31/1
  Constant67/1 -> RelationalOperator43/2
  Constant66/1 -> RelationalOperator43/1
  RelationalOperator43/1 -> LogicalOperator20/1
  Constant70/1 -> RelationalOperator44/2
  Constant68/1 -> RelationalOperator44/1
  RelationalOperator44/1 -> LogicalOperator22/1
  Constant61/1 -> RelationalOperator47/1
  Constant71/1 -> RelationalOperator47/2
  RelationalOperator47/1 -> LogicalOperator25/1
  Constant69/1 -> RelationalOperator46/1
  Constant72/1 -> RelationalOperator46/2
  RelationalOperator46/1 -> LogicalOperator27/1
  Logical Operator21/1 -> LogicalOperator20/2
  Logical Operator30/1 -> LogicalOperator31/2
  RelationalOperator2/1 -> Logical Operator29/2, Logical Operator20/2, Logical Operator28/2, Logical Operator27/2, Logical Operator22/2, Logical Operator26/2, Logical Operator25/2, Logical Operator23/2, Logical Operator21/2, Logical Operator30/2
  Constant89/1 -> RelationalOperator58/2
  RelationalOperator58/1 -> Logical Operator21/1
  Constant86/1 -> RelationalOperator57/2
  Constant87/1 -> RelationalOperator59/2
  RelationalOperator59/1 -> Logical Operator25/1
  Logical Operator25/1 -> LogicalOperator27/2
  Logical Operator23/1 -> LogicalOperator25/2
  Constant88/1 -> RelationalOperator62/2
  Logical Operator26/1 -> LogicalOperator22/2
  RelationalOperator63/1 -> Logical Operator30/1
  RelationalOperator57/1 -> Logical Operator23/1
  RelationalOperator62/1 -> Logical Operator26/1
  [Number_Subfunction] -> RelationalOperator63/1
  [Number_Subfunction] -> RelationalOperator58/1
  [Number_Subfunction] -> RelationalOperator62/1
  [Number_Subfunction] -> RelationalOperator57/1
  [Number_Subfunction] -> RelationalOperator59/1
  Constant91/1 -> RelationalOperator60/1
  Constant75/1 -> RelationalOperator60/2
  RelationalOperator60/1 -> LogicalOperator23/1
  Constant93/1 -> RelationalOperator61/2
  RelationalOperator61/1 -> Logical Operator22/1
  Logical Operator22/1 -> LogicalOperator23/2
  [Number_Subfunction] -> RelationalOperator61/1
  Constant85/1 -> RelationalOperator56/2
  Constant73/1 -> RelationalOperator64/1
  Constant77/1 -> RelationalOperator64/2
  RelationalOperator64/1 -> LogicalOperator26/1
  Constant79/1 -> RelationalOperator48/2
  Constant78/1 -> RelationalOperator48/1
  RelationalOperator48/1 -> LogicalOperator29/1
  Constant81/1 -> RelationalOperator49/2
  Constant80/1 -> RelationalOperator49/1
  RelationalOperator49/1 -> LogicalOperator30/1
  Constant94/1 -> RelationalOperator50/1
  Constant92/1 -> RelationalOperator50/2
  RelationalOperator50/1 -> LogicalOperator21/1
  Logical Operator28/1 -> LogicalOperator29/2
  Logical Operator27/1 -> LogicalOperator26/2
  Constant84/1 -> RelationalOperator54/2
  RelationalOperator54/1 -> Logical Operator28/1
  Constant82/1 -> RelationalOperator53/2
  Logical Operator29/1 -> LogicalOperator21/2
  Constant83/1 -> RelationalOperator55/2
  Logical Operator20/1 -> LogicalOperator30/2
  RelationalOperator56/1 -> Logical Operator27/1
  RelationalOperator53/1 -> Logical Operator29/1
  RelationalOperator55/1 -> Logical Operator20/1
  [Number_Subfunction] -> RelationalOperator56/1
  [Number_Subfunction] -> RelationalOperator54/1
  [Number_Subfunction] -> RelationalOperator55/1
  [Number_Subfunction] -> RelationalOperator53/1
  Constant120/1 -> RelationalOperator83/2
  Constant97/1 -> RelationalOperator65/1
  Constant96/1 -> RelationalOperator65/2
  RelationalOperator65/1 -> LogicalOperator41/1
  Constant98/1 -> RelationalOperator66/2
  Constant99/1 -> RelationalOperator66/1
  RelationalOperator66/1 -> LogicalOperator32/1
  Constant100/1 -> RelationalOperator67/2
  Constant101/1 -> RelationalOperator67/1
  RelationalOperator67/1 -> LogicalOperator34/1
  Constant95/1 -> RelationalOperator69/1
  Constant103/1 -> RelationalOperator69/2
  RelationalOperator69/1 -> LogicalOperator36/1
  Constant102/1 -> RelationalOperator68/1
  Constant104/1 -> RelationalOperator68/2
  RelationalOperator68/1 -> LogicalOperator38/1
  Logical Operator32/1 -> LogicalOperator32/2
  Logical Operator40/1 -> LogicalOperator41/2
  RelationalOperator3/1 -> Logical Operator39/2, Logical Operator31/2, Logical Operator38/2, Logical Operator37/2, Logical Operator33/2, Logical Operator36/2, Logical Operator35/2, Logical Operator34/2, Logical Operator32/2, Logical Operator40/2
  Constant119/1 -> RelationalOperator78/2
  RelationalOperator78/1 -> Logical Operator32/1
  Constant116/1 -> RelationalOperator77/2
  Constant117/1 -> RelationalOperator79/2
  RelationalOperator79/1 -> Logical Operator35/1
  Logical Operator35/1 -> LogicalOperator38/2
  Logical Operator34/1 -> LogicalOperator36/2
  Constant118/1 -> RelationalOperator82/2
  Logical Operator36/1 -> LogicalOperator34/2
  RelationalOperator83/1 -> Logical Operator40/1
  RelationalOperator77/1 -> Logical Operator34/1
  RelationalOperator82/1 -> Logical Operator36/1
  [Number_Subfunction] -> RelationalOperator83/1
  [Number_Subfunction] -> RelationalOperator78/1
  [Number_Subfunction] -> RelationalOperator82/1
  [Number_Subfunction] -> RelationalOperator77/1
  [Number_Subfunction] -> RelationalOperator79/1
  Constant121/1 -> RelationalOperator80/1
  Constant105/1 -> RelationalOperator80/2
  RelationalOperator80/1 -> LogicalOperator35/1
  Constant123/1 -> RelationalOperator81/2
  RelationalOperator81/1 -> Logical Operator33/1
  Logical Operator33/1 -> LogicalOperator35/2
  [Number_Subfunction] -> RelationalOperator81/1
  Constant115/1 -> RelationalOperator76/2
  Constant106/1 -> RelationalOperator84/1
  Constant107/1 -> RelationalOperator84/2
  RelationalOperator84/1 -> LogicalOperator37/1
  Constant109/1 -> RelationalOperator70/2
  Constant108/1 -> RelationalOperator70/1
  RelationalOperator70/1 -> LogicalOperator39/1
  Constant111/1 -> RelationalOperator71/2
  Constant110/1 -> RelationalOperator71/1
  RelationalOperator71/1 -> LogicalOperator40/1
  Constant124/1 -> RelationalOperator72/1
  Constant122/1 -> RelationalOperator72/2
  RelationalOperator72/1 -> LogicalOperator33/1
  Logical Operator38/1 -> LogicalOperator39/2
  Logical Operator37/1 -> LogicalOperator37/2
  Constant114/1 -> RelationalOperator74/2
  RelationalOperator74/1 -> Logical Operator38/1
  Constant112/1 -> RelationalOperator73/2
  Logical Operator39/1 -> LogicalOperator33/2
  Constant113/1 -> RelationalOperator75/2
  Logical Operator31/1 -> LogicalOperator40/2
  RelationalOperator76/1 -> Logical Operator37/1
  RelationalOperator73/1 -> Logical Operator39/1
  RelationalOperator75/1 -> Logical Operator31/1
  [Number_Subfunction] -> RelationalOperator76/1
  [Number_Subfunction] -> RelationalOperator74/1
  [Number_Subfunction] -> RelationalOperator75/1
  [Number_Subfunction] -> RelationalOperator73/1
  LogicalOperator31/1 -> LogicalOperator43/1
  LogicalOperator20/1 -> LogicalOperator43/2
  LogicalOperator22/1 -> LogicalOperator43/3
  LogicalOperator25/1 -> LogicalOperator43/4
  LogicalOperator27/1 -> LogicalOperator43/5
  LogicalOperator23/1 -> LogicalOperator43/6
  LogicalOperator26/1 -> LogicalOperator43/7
  LogicalOperator29/1 -> LogicalOperator43/8
  LogicalOperator30/1 -> LogicalOperator43/9
  LogicalOperator21/1 -> LogicalOperator43/10
  LogicalOperator41/1 -> LogicalOperator44/1
  LogicalOperator32/1 -> LogicalOperator44/2
  LogicalOperator34/1 -> LogicalOperator44/3
  LogicalOperator36/1 -> LogicalOperator44/4
  LogicalOperator38/1 -> LogicalOperator44/5
  LogicalOperator35/1 -> LogicalOperator44/6
  LogicalOperator37/1 -> LogicalOperator44/7
  LogicalOperator39/1 -> LogicalOperator44/8
  LogicalOperator40/1 -> LogicalOperator44/9
  LogicalOperator33/1 -> LogicalOperator44/10
  LogicalOperator9/1 -> LogicalOperator42/1
  LogicalOperator11/1 -> LogicalOperator42/2
  LogicalOperator13/1 -> LogicalOperator42/3
  LogicalOperator15/1 -> LogicalOperator42/4
  LogicalOperator17/1 -> LogicalOperator42/5
  LogicalOperator14/1 -> LogicalOperator42/6
  LogicalOperator16/1 -> LogicalOperator42/7
  LogicalOperator18/1 -> LogicalOperator42/8
  LogicalOperator19/1 -> LogicalOperator42/9
  LogicalOperator12/1 -> LogicalOperator42/10
  LogicalOperator4/1 -> LogicalOperator24/1
  LogicalOperator42/1 -> LogicalOperator24/2
  LogicalOperator43/1 -> LogicalOperator24/3
  LogicalOperator44/1 -> LogicalOperator24/4
